simulink model "TosAeb"  (R2021a)
settings: Solver=FixedStepDiscrete  StartTime=0.0  StopTime=inf  FixedStep=0.01  RelTol=1e-3
undefined symbols (not defined in the file or in its folder): ACCModSts, AccSts1, AEB_ObjectMovingOrientation, AEB_ObjectMovingState, CN_BTCActive, CN_CurrentGearR, CN_CurrentGearR_DHT, CN_PTCActive, CN_VDCActive, CN_VSC_EspOff, CN_VSC_FCW_AEB_Error, CN_VSC_FCW_AEB_NoDispay, CN_VSC_FCW_AEB_PerfmDgdtn, CN_VSC_FCW_AEB_TempUnAvlbl, CN_VSC_VehStandstill, CNaccelAvoidMax, CNaccelAvoidMin, CNbrakePedalPress, CNcomfortStrLeftAccelMax, CNcomfortStrLeftAccelMin, CNcomfortStrRightAccelMax, CNcomfortStrRightAccelMin, CNDrvDoorOpen, CNDrvModeEco, CNDrvModeNorm, CNDrvModeOther, CNDrvModeSport, CNDrvSBRreminder, CNNull, CNobjInvalidID, CNPassDoorOpen, CNPassSBRreminder, CNPEBSAccelNegInf, CNPEBSAccelNull, CNPEBSTimeInf, CNPEBSTimeNull, CNSTOPMAX, CNSTOPNULL, CNsubjWidth, CNSwtOFF, CNSwtON, CNTTCMAX, CNTTCNULL, CNVehConfigAEBCycNone, CNVehConfigAEBNone, CNVehConfigAEBPedNone, CNVehConfigFCWNone, CNVehConfigJANone, CollisionSeverity, FALSE, FCWSnvty, JACycObjCalcInfoDefaultParams, JAPedObjCalcInfoDefaultParams, JAVehObjCalcInfoDefaultParams, latCycObjCalcInfoDefaultParams, latPedObjCalcInfoDefaultParams, longObjCalcInfoDefaultParams, ModSts, movingStatus, ObjectType ... (+1112)
libraries: simulink x3031, AEBSFunctionLib x50, Ta_Library x33, AEBSStateMachineLib x23, Mylibrary x12, simulink_extras x7, ObjSelect_lib x3, dspstat3 x2
(part 20 of 102)

== system TosAeb/FcwAebJa_Step_sys/ALG_AEBSystem/ALG_AEBOutput/AEBS_DVRInfoCalc/AEB_LATCYC_DVR_INFO/Subsystem ==
  latCycTgtCalcInfo : Inport
  Action Port : ActionPort  ActionPortLabel=else
  AEBObjStateMap : Subsystem
  Bus Creator : BusCreator  Inputs=12
  Bus Selector2 : BusSelector  OutputSignals=latCycObjCalcInfo.latCycObjRawInfo.ObjectID,latCycObjCalcInfo.latCycObjRawInfo.ObjectTypeCameraConfirmed,latCycObjCalcInfo.latCycObjRawInfo.ObjectMovingState,latCycObjCalcInfo.latCycObjRawInfo.ObjectMovingOrientation,latCycObjCalcInfo.latCycObjRawInfo.dx,latCycObjCalcInfo.latCycObjRawInfo.dy,latCycObjCalcInfo.latCycObjRawInfo.vx,latCycObjCalcInfo.latCycObjRawInfo.vy,latCycObjCalcInfo.latCycObjRawInfo.ax,latCycObjCalcInfo.latCycObjRawInfo.ay,latCycObjCalcInfo.latCycObjRawInfo.ObjectExistProb,latCycObjCalcInfo.latCycObjRawInfo.ObjectIsObstacleProb,latCycObjCalcInfo.latCycObjRawInfo.ObjectLifeCycle
  Signal Conversion{26..37} (12 alike) : SignalConversion  OverrideOpt=off
  AEB_LATCYC_DVR_INFO : Outport
  wires:
  Signal Conversion30/1 -> Bus Creator/5  "CyclistObjDy"
  Signal Conversion28/1 -> Bus Creator/3  "CyclistObjState"
  Signal Conversion26/1 -> Bus Creator/1  "CyclistObjID"
  Signal Conversion36/1 -> Bus Creator/11  "CyclistObjObstcl"
  Signal Conversion35/1 -> Bus Creator/10  "CyclistObjExist"
  Bus Selector2/3 -> AEBObjStateMap/1(ObjectMovingState)  "<ObjectMovingState>"
  Bus Selector2/4 -> AEBObjStateMap/2(ObjectMovingOrientation)  "<ObjectMovingOrientation>"
  Signal Conversion33/1 -> Bus Creator/8  "CyclistObjAx"
  AEBObjStateMap/1(AEBObjState) -> Signal Conversion28/1
  Bus Selector2/5 -> Signal Conversion29/1  "<dx>"
  Bus Selector2/6 -> Signal Conversion30/1  "<dy>"
  latCycTgtCalcInfo/1 -> Bus Selector2/1
  Bus Selector2/7 -> Signal Conversion31/1  "<vx>"
  Bus Selector2/8 -> Signal Conversion32/1  "<vy>"
  Bus Selector2/9 -> Signal Conversion33/1  "<ax>"
  Bus Selector2/12 -> Signal Conversion36/1  "<ObjectIsObstacleProb>"
  Bus Selector2/1 -> Signal Conversion26/1  "<ObjectID>"
  Signal Conversion31/1 -> Bus Creator/6  "CyclistObjVx"
  Bus Creator/1 -> AEB_LATCYC_DVR_INFO/1
  Signal Conversion29/1 -> Bus Creator/4  "CyclistObjDx"
  Bus Selector2/11 -> Signal Conversion35/1  "<ObjectExistProb>"
  Signal Conversion37/1 -> Bus Creator/12  "CyclistObjCntAlive"
  Bus Selector2/2 -> Signal Conversion27/1  "<ObjectTypeCameraConfirmed>"
  Signal Conversion27/1 -> Bus Creator/2  "CyclistObjVideoCfmd"
  Bus Selector2/13 -> Signal Conversion37/1  "<ObjectLifeCycle>"
  Bus Selector2/10 -> Signal Conversion34/1  "<ay>"
  Signal Conversion34/1 -> Bus Creator/9  "CyclistObjAy"
  Signal Conversion32/1 -> Bus Creator/7  "CyclistObjVy"

== system TosAeb/FcwAebJa_Step_sys/ALG_AEBSystem/ALG_AEBOutput/AEBS_DVRInfoCalc/AEB_LATPED_DVR_INFO/If Action Subsystem ==
  Action Port : ActionPort  ActionPortLabel=if(u1)
  Bus Creator : BusCreator  Inputs=12
  Constant{,1..11} (12 alike) : Constant  Value=0
  Data Type Conversion{,1..11} (12 alike) : DataTypeConversion
  AEB_LATPED_DVR_INFO : Outport
  wires:
  Constant8/1 -> Data Type Conversion8/1
  Constant9/1 -> Data Type Conversion9/1
  Constant11/1 -> Data Type Conversion11/1
  Constant10/1 -> Data Type Conversion10/1
  Constant4/1 -> Data Type Conversion4/1
  Constant5/1 -> Data Type Conversion5/1
  Constant7/1 -> Data Type Conversion7/1
  Constant6/1 -> Data Type Conversion6/1
  Constant2/1 -> Data Type Conversion2/1
  Constant3/1 -> Data Type Conversion3/1
  Constant1/1 -> Data Type Conversion1/1
  Constant/1 -> Data Type Conversion/1
  Data Type Conversion6/1 -> Bus Creator/7  "PedObjVy"
  Data Type Conversion1/1 -> Bus Creator/2  "PedObjVideoCfmd"
  Bus Creator/1 -> AEB_LATPED_DVR_INFO/1
  Data Type Conversion2/1 -> Bus Creator/3  "PedObjState"
  Data Type Conversion11/1 -> Bus Creator/12  "PedObjCntAlive"
  Data Type Conversion4/1 -> Bus Creator/5  "PedObjDy"
  Data Type Conversion7/1 -> Bus Creator/8  "PedObjAx"
  Data Type Conversion3/1 -> Bus Creator/4  "PedObjDx"
  Data Type Conversion/1 -> Bus Creator/1  "PedObjID"
  Data Type Conversion10/1 -> Bus Creator/11  "PedObjObstcl"
  Data Type Conversion8/1 -> Bus Creator/9  "PedObjAy"
  Data Type Conversion9/1 -> Bus Creator/10  "PedObjExist"
  Data Type Conversion5/1 -> Bus Creator/6  "PedObjVx"

== system TosAeb/FcwAebJa_Step_sys/ALG_AEBSystem/ALG_AEBOutput/AEBS_DVRInfoCalc/AEB_LATPED_DVR_INFO/Subsystem ==
  latPedTgtCalcInfo : Inport
  Action Port : ActionPort  ActionPortLabel=else
  AEBObjStateMap : Subsystem
  Bus Creator : BusCreator  Inputs=12
  Bus Selector1 : BusSelector  OutputSignals=latPedObjCalcInfo.latPedObjRawInfo.ObjectID,latPedObjCalcInfo.latPedObjRawInfo.ObjectTypeCameraConfirmed,latPedObjCalcInfo.latPedObjRawInfo.ObjectMovingState,latPedObjCalcInfo.latPedObjRawInfo.ObjectMovingOrientation,latPedObjCalcInfo.latPedObjRawInfo.dx,latPedObjCalcInfo.latPedObjRawInfo.dy,latPedObjCalcInfo.latPedObjRawInfo.vx,latPedObjCalcInfo.latPedObjRawInfo.vy,latPedObjCalcInfo.latPedObjRawInfo.ax,latPedObjCalcInfo.latPedObjRawInfo.ay,latPedObjCalcInfo.latPedObjRawInfo.ObjectExistProb,latPedObjCalcInfo.latPedObjRawInfo.ObjectIsObstacleProb,latPedObjCalcInfo.latPedObjRawInfo.ObjectLifeCycle
  Signal Conversion{14..25} (12 alike) : SignalConversion  OverrideOpt=off
  AEB_LATPED_DVR_INFO : Outport
  wires:
  Bus Selector1/6 -> Signal Conversion18/1  "<dy>"
  Bus Selector1/7 -> Signal Conversion19/1  "<vx>"
  Bus Selector1/2 -> Signal Conversion15/1  "<ObjectTypeCameraConfirmed>"
  Bus Selector1/3 -> AEBObjStateMap/1(ObjectMovingState)  "<ObjectMovingState>"
  Bus Selector1/8 -> Signal Conversion20/1  "<vy>"
  Bus Selector1/10 -> Signal Conversion22/1  "<ay>"
  Bus Selector1/9 -> Signal Conversion21/1  "<ax>"
  Bus Selector1/4 -> AEBObjStateMap/2(ObjectMovingOrientation)  "<ObjectMovingOrientation>"
  Bus Creator/1 -> AEB_LATPED_DVR_INFO/1
  Signal Conversion20/1 -> Bus Creator/7  "PedObjVy"
  Signal Conversion19/1 -> Bus Creator/6  "PedObjVx"
  Signal Conversion23/1 -> Bus Creator/10  "PedObjExist"
  Bus Selector1/13 -> Signal Conversion25/1  "<ObjectLifeCycle>"
  Signal Conversion21/1 -> Bus Creator/8  "PedObjAx"
  Bus Selector1/11 -> Signal Conversion23/1  "<ObjectExistProb>"
  Signal Conversion22/1 -> Bus Creator/9  "PedObjAy"
  Signal Conversion24/1 -> Bus Creator/11  "PedObjObstcl"
  Signal Conversion14/1 -> Bus Creator/1  "PedObjID"
  Signal Conversion15/1 -> Bus Creator/2  "PedObjVideoCfmd"
  latPedTgtCalcInfo/1 -> Bus Selector1/1
  Bus Selector1/12 -> Signal Conversion24/1  "<ObjectIsObstacleProb>"
  Signal Conversion25/1 -> Bus Creator/12  "PedObjCntAlive"
  Signal Conversion17/1 -> Bus Creator/4  "PedObjDx"
  AEBObjStateMap/1(AEBObjState) -> Signal Conversion16/1
  Signal Conversion18/1 -> Bus Creator/5  "PedObjDy"
  Bus Selector1/5 -> Signal Conversion17/1  "<dx>"
  Signal Conversion16/1 -> Bus Creator/3  "PedObjState"
  Bus Selector1/1 -> Signal Conversion14/1  "<ObjectID>"

== system TosAeb/FcwAebJa_Step_sys/ALG_AEBSystem/ALG_AEBOutput/AEBS_DVRInfoCalc/AEB_LON_DVR_INFO/If Action Subsystem ==
  longObjCalcInfo : Inport
  Action Port : ActionPort  ActionPortLabel=else
  AEBObjStateMap : Subsystem
  Bus Creator : BusCreator  Inputs=14
  Bus Selector : BusSelector  OutputSignals=LongObjRawInfo.ObjectID,LongObjRawInfo.ObjectTypeCameraConfirmed,LongObjRawInfo.ObjectMovingState,LongObjRawInfo.ObjectMovingOrientation,LongObjRawInfo.dx,LongObjRawInfo.dy,LongObjRawInfo.vx,LongObjRawInfo.vy,LongObjRawInfo.ax,LongObjRawInfo.ay,LongObjRawInfo.ObjectExistProb,LongObjRawInfo.ObjectIsObstacleProb,LongObjRawInfo.ObjectLifeCycle,overlapRatio,probEscape
  Signal Conversion{,1..13} (14 alike) : SignalConversion  OverrideOpt=off
  AEB_LON_DVR_INFO : Outport
  wires:
  Bus Creator/1 -> AEB_LON_DVR_INFO/1
  Bus Selector/6 -> Signal Conversion4/1  "<dy>"
  Signal Conversion3/1 -> Bus Creator/4  "AEBObjDx"
  Signal Conversion/1 -> Bus Creator/1  "AEBObjID"
  longObjCalcInfo/1 -> Bus Selector/1
  Signal Conversion10/1 -> Bus Creator/11  "AEBObjObstcl"
  Signal Conversion7/1 -> Bus Creator/8  "AEBObjAx"
  Bus Selector/13 -> Signal Conversion11/1  "<ObjectLifeCycle>"
  Bus Selector/15 -> Signal Conversion13/1  "<probEscape>"
  AEBObjStateMap/1(AEBObjState) -> Signal Conversion2/1
  Bus Selector/8 -> Signal Conversion6/1  "<vy>"
  Signal Conversion2/1 -> Bus Creator/3  "AEBObjState"
  Signal Conversion8/1 -> Bus Creator/9  "AEBObjAy"
  Bus Selector/14 -> Signal Conversion12/1  "<overlapRatio>"
  Bus Selector/3 -> AEBObjStateMap/1(ObjectMovingState)  "<ObjectMovingState>"
  Bus Selector/2 -> Signal Conversion1/1  "<ObjectTypeCameraConfirmed>"
  Signal Conversion9/1 -> Bus Creator/10  "AEBObjExist"
  Bus Selector/1 -> Signal Conversion/1  "<ObjectID>"
  Signal Conversion13/1 -> Bus Creator/14  "AEBObjDrvby"
  Signal Conversion5/1 -> Bus Creator/6  "AEBObjVx"
  Signal Conversion11/1 -> Bus Creator/12  "AEBObjCntAlive"
  Bus Selector/5 -> Signal Conversion3/1  "<dx>"
  Signal Conversion12/1 -> Bus Creator/13  "AEBObjOverlap"
  Signal Conversion1/1 -> Bus Creator/2  "AEBObjVideoCfmd"
  Signal Conversion6/1 -> Bus Creator/7  "AEBObjVy"
  Signal Conversion4/1 -> Bus Creator/5  "AEBObjDy"
  Bus Selector/10 -> Signal Conversion8/1  "<ay>"
  Bus Selector/4 -> AEBObjStateMap/2(ObjectMovingOrientation)  "<ObjectMovingOrientation>"
  Bus Selector/7 -> Signal Conversion5/1  "<vx>"
  Bus Selector/12 -> Signal Conversion10/1  "<ObjectIsObstacleProb>"
  Bus Selector/9 -> Signal Conversion7/1  "<ax>"
  Bus Selector/11 -> Signal Conversion9/1  "<ObjectExistProb>"

== system TosAeb/FcwAebJa_Step_sys/ALG_AEBSystem/ALG_AEBOutput/AEBS_DVRInfoCalc/AEB_LON_DVR_INFO/If Action Subsystem1 ==
  Action Port : ActionPort  ActionPortLabel=if(u1)
  Bus Creator : BusCreator  Inputs=14
  Constant{,1..13} (14 alike) : Constant  Value=0
  Data Type Conversion{,1..13} (14 alike) : DataTypeConversion
  AEB_LON_DVR_INFO : Outport
  wires:
  Constant12/1 -> Data Type Conversion12/1
  Constant13/1 -> Data Type Conversion13/1
  Constant7/1 -> Data Type Conversion7/1
  Constant8/1 -> Data Type Conversion8/1
  Constant11/1 -> Data Type Conversion11/1
  Constant10/1 -> Data Type Conversion10/1
  Constant9/1 -> Data Type Conversion9/1
  Constant4/1 -> Data Type Conversion4/1
  Constant5/1 -> Data Type Conversion5/1
  Constant6/1 -> Data Type Conversion6/1
  Constant3/1 -> Data Type Conversion3/1
  Constant2/1 -> Data Type Conversion2/1
  Constant1/1 -> Data Type Conversion1/1
  Constant/1 -> Data Type Conversion/1
  Data Type Conversion6/1 -> Bus Creator/7  "AEBObjVy"
  Data Type Conversion4/1 -> Bus Creator/5  "AEBObjDy"
  Data Type Conversion1/1 -> Bus Creator/2  "AEBObjVideoCfmd"
  Data Type Conversion5/1 -> Bus Creator/6  "AEBObjVx"
  Data Type Conversion11/1 -> Bus Creator/12  "AEBObjCntAlive"
  Data Type Conversion12/1 -> Bus Creator/13  "AEBObjOverlap"
  Data Type Conversion13/1 -> Bus Creator/14  "AEBObjDrvby"
  Data Type Conversion2/1 -> Bus Creator/3  "AEBObjState"
  Data Type Conversion9/1 -> Bus Creator/10  "AEBObjExist"
  Data Type Conversion8/1 -> Bus Creator/9  "AEBObjAy"
  Data Type Conversion7/1 -> Bus Creator/8  "AEBObjAx"
  Data Type Conversion10/1 -> Bus Creator/11  "AEBObjObstcl"
  Data Type Conversion3/1 -> Bus Creator/4  "AEBObjDx"
  Data Type Conversion/1 -> Bus Creator/1  "AEBObjID"
  Bus Creator/1 -> AEB_LON_DVR_INFO/1

== system TosAeb/FcwAebJa_Step_sys/ALG_AEBSystem/ALG_AEBOutput/AEBS_IPHUTInfoCalc/AR_HUD_InfoMap/AEB_Cyc_collisionprob ==
  cycPrefillFuncImpArb : Inport
  cycAEBpartFuncImpArb : Inport  Port=2
  cycAEBfullFuncImpArb : Inport  Port=3
  Compare To Constant5 : simulink/Logic and Bit Operations/Compare To Constant  relop===  const=ModSts.ActiveState  OutDataTypeStr=boolean
  Compare To Constant6 : simulink/Logic and Bit Operations/Compare To Constant  relop===  const=ModSts.ActiveState  OutDataTypeStr=boolean
  Compare To Constant7 : simulink/Logic and Bit Operations/Compare To Constant  relop===  const=ModSts.ActiveState  OutDataTypeStr=boolean
  Logical Operator1 : Logic  Operator=OR  Inputs=3  AllPortsSameDT=off  OutDataTypeStr=boolean
  AEB_Cyc_collisionprob : Outport
  wires:
  Compare To Constant5/1 -> Logical Operator1/1
  cycAEBfullFuncImpArb/1 -> Compare To Constant7/1
  cycAEBpartFuncImpArb/1 -> Compare To Constant6/1
  Compare To Constant6/1 -> Logical Operator1/2
  cycPrefillFuncImpArb/1 -> Compare To Constant5/1
  Logical Operator1/1 -> AEB_Cyc_collisionprob/1
  Compare To Constant7/1 -> Logical Operator1/3

== system TosAeb/FcwAebJa_Step_sys/ALG_AEBSystem/ALG_AEBOutput/AEBS_IPHUTInfoCalc/AR_HUD_InfoMap/AEB_Ped_collisionprob ==
  pedPreWarningFuncImpArb : Inport
  pedPrefillFuncImpArb : Inport  Port=2
  pedABAFuncImpArb : Inport  Port=3
  pedAEBfullFuncImpArb : Inport  Port=4
  Compare To Constant{1..4} (4 alike) : simulink/Logic and Bit Operations/Compare To Constant  relop===  const=ModSts.ActiveState  OutDataTypeStr=boolean
  Logical Operator : Logic  Operator=OR  Inputs=4  AllPortsSameDT=off  OutDataTypeStr=boolean
  AEB_Ped_collisionprob : Outport
  wires:
  pedABAFuncImpArb/1 -> Compare To Constant2/1
  pedPrefillFuncImpArb/1 -> Compare To Constant1/1
  Compare To Constant3/1 -> Logical Operator/4
  Compare To Constant1/1 -> Logical Operator/2
  pedAEBfullFuncImpArb/1 -> Compare To Constant3/1
  pedPreWarningFuncImpArb/1 -> Compare To Constant4/1
  Logical Operator/1 -> AEB_Ped_collisionprob/1
  Compare To Constant4/1 -> Logical Operator/1
  Compare To Constant2/1 -> Logical Operator/3

== system TosAeb/AEB_Objsel/TA_InfoRebuild/TAObjInfoCalc/For Each Subsystem/Info_rebuild/Road_relative/LineInfo_Process ==
  Road_Info : Inport
  Bus Selector : BusSelector  OutputSignals=lineData
  LineInfo_Process_Left : Subsystem
  LineInfo_Process_Right : Subsystem
  Selector : Selector  IndexMode=Zero-based  InputPortWidth=4  IndexOptions=Index vector (dialog)  Indices=[0]  OutputSizes=1
  Selector1 : Selector  IndexMode=Zero-based  InputPortWidth=4  IndexOptions=Index vector (dialog)  Indices=[1]  OutputSizes=1
  LeftLine_Info : Outport
  RightLine_Info : Outport  Port=2
  wires:
  Bus Selector/1 -> Selector1/1, Selector/1  "<lineData>"
  LineInfo_Process_Left/1(LeftLine_Info) -> LeftLine_Info/1
  Selector/1 -> LineInfo_Process_Left/1(Leftline_Data)
  Road_Info/1 -> Bus Selector/1
  LineInfo_Process_Right/1(RightLine_Info) -> RightLine_Info/1
  Selector1/1 -> LineInfo_Process_Right/1(Rightline_Data)

== system TosAeb/AEB_Objsel/TA_InfoRebuild/TAObjInfoCalc/For Each Subsystem/Info_rebuild/Road_relative/RebuildInfo_Process ==
  Obj_Infomation : Inport
  LeftLine_Info : Inport  Port=2
  RightLine_Info : Inport  Port=3
  If : If
  If Action Subsystem : Subsystem  TreatAsAtomicUnit=on
  If Action Subsystem1 : Subsystem  TreatAsAtomicUnit=on
  Merge : Merge
  Merge1 : Merge
  Merge2 : Merge
  Subsystem : Subsystem
  objLatDist2LaneCenter : Outport
  objLatDist2EgoCenter : Outport  Port=2
  objPosition : Outport  Port=3
  wires:
  Subsystem/1(if_judge) -> If/1
  If/1 -> If Action Subsystem/Action
  If/2 -> If Action Subsystem1/Action
  Obj_Infomation/1 -> If Action Subsystem1/1(Obj_Infomation), Subsystem/1(Obj_Infomation)
  LeftLine_Info/1 -> If Action Subsystem1/2(LeftLine_Info)
  RightLine_Info/1 -> If Action Subsystem1/3(RightLine_Info)
  If Action Subsystem/1(objLatDist2LaneCenter) -> Merge/1
  Merge/1 -> objLatDist2LaneCenter/1
  If Action Subsystem1/1(objLatDist2LaneCenter) -> Merge/2
  If Action Subsystem/2(objLatDist2EgoCenter) -> Merge1/1
  If Action Subsystem1/2(objLatDist2EgoCenter) -> Merge1/2
  If Action Subsystem/3(objPosition) -> Merge2/1
  If Action Subsystem1/3(objPosition) -> Merge2/2
  Merge1/1 -> objLatDist2EgoCenter/1
  Merge2/1 -> objPosition/1

== system TosAeb/AEB_Objsel/TA_Processor/AEB_ObjSelect/TargetInfoCheck/Data_precess/TA_EffectCalculate/Obj_FilterFlgCalculate ==
  Utility : Inport
  Overlap_Current : Inport  Port=2
  Overlap_Prediction : Inport  Port=3
  PahEstimn : Inport  Port=4
  TA_ObjInfo : Inport  Port=5
  VehSelfData : Inport  Port=6
  JA_ObjFilter : Subsystem
  Lat_ObjFilter : Subsystem
  Long_ObjFilter : Subsystem
  WeightCoef_Cal : Subsystem
  Overlap_Flg : Outport
  Boudingbox_Calculate : Outport  Port=2
  Obj_Coef : Outport  Port=3
  TTI : Outport  Port=4
  LatObj_Valid : Outport  Port=5
  Lat_ObjIntersect : Outport  Port=6
  Lat_TTR : Outport  Port=7
  JA_TTR : Outport  Port=8
  Meas_WeightCoef_1 : Outport  Port=9
  Meas_WeightCoef_2 : Outport  Port=10
  wires:
  TA_ObjInfo/1 -> [TA_ObjInfo], Long_ObjFilter/2(TA_ObjInfo)
  VehSelfData/1 -> [VehSelfData], Long_ObjFilter/3(VehSelfData)
  Utility/1 -> [Utility], WeightCoef_Cal/2(Utility)
  Overlap_Current/1 -> Overlap_Flg/1, WeightCoef_Cal/1(Overlap_Current)  "Overlap_Current"
  Overlap_Prediction/1 -> WeightCoef_Cal/3(Overlap_Prediction)  "Overlap_Prediction"
  PahEstimn/1 -> [PahEstimn], Long_ObjFilter/4(" PahEstimn")
  Long_ObjFilter/1(Boudingbox_Calculate) -> Boudingbox_Calculate/1
  Long_ObjFilter/2(Obj_Coef) -> Obj_Coef/1
  Long_ObjFilter/3(TTI) -> TTI/1
  [VehSelfData] -> WeightCoef_Cal/4(VehSelfData)
  [TA_ObjInfo] -> Lat_ObjFilter/2(TA_ObjInfo)
  Lat_ObjFilter/3(Lat_TTR) -> Lat_TTR/1
  Lat_ObjFilter/1(LatObj_Valid) -> LatObj_Valid/1
  Lat_ObjFilter/2(Lat_ObjIntersect) -> Lat_ObjIntersect/1
  [Utility] -> Lat_ObjFilter/1(Utility)
  [VehSelfData] -> Lat_ObjFilter/3(VehSelfData)
  [TA_ObjInfo] -> JA_ObjFilter/1(TA_ObjInfo)
  [PahEstimn] -> JA_ObjFilter/2(PahEstimn)
  [VehSelfData] -> JA_ObjFilter/3(VehSelfData)
  JA_ObjFilter/1(ObjColliInfo) -> JA_TTR/1
  WeightCoef_Cal/1(Obj_WeightCoef) -> Meas_WeightCoef_1/1, Long_ObjFilter/1(Obj_WeightCoef)
  Long_ObjFilter/4(Long_ObjWeightCoef) -> Meas_WeightCoef_2/1

== system TosAeb/AEB_Objsel/TA_Processor/AEB_ObjSelect/TargetInfoCheck/Data_precess/TA_EffectCalculate/ValideateObj ==
  TA_ObsInfo : Inport
  VehSelfData : Inport  Port=2
  PathEstimn : Inport  Port=3
  ObjPos_Calculate : Subsystem
  ValidObj_PreProcess : Subsystem
  Utility : Outport
  Overlap_Current : Outport  Port=2
  Overlap_Prediction : Outport  Port=3
  wires:
  VehSelfData/1 -> ValidObj_PreProcess/2(VehSelfData)
  ObjPos_Calculate/2(Overlap_Current) -> Overlap_Current/1
  ObjPos_Calculate/3(Overlap_Prediction) -> Overlap_Prediction/1
  TA_ObsInfo/1 -> ValidObj_PreProcess/1(TA_ObjInfo), ObjPos_Calculate/1(TA_ObsInfo)
  PathEstimn/1 -> ObjPos_Calculate/3(PathEstimn)
  ValidObj_PreProcess/1(Obj_Enable) -> ObjPos_Calculate/2(Obj_Enable)
  ObjPos_Calculate/1(Utility) -> Utility/1

== system TosAeb/AEB_Objsel/TA_Processor/AEB_ObjSelect/TargetSelect/Lat_ObjSelect/AEB_LatObj/AEB_LatTAelse ==
  LatObj_Valid : Inport
  TA_ObjInfo : Inport  Port=2
  Lat_ObjIntersect : Inport  Port=3
  Lat_TTR : Inport  Port=4
  Action Port : ActionPort  ActionPortLabel=else
  Bus Selector : BusSelector  OutputSignals=TA_ObjID
  Constant : Constant  Value=zeros(10,1)  OutDataTypeStr=uint8
  Constant1 : Constant  Value=zeros(10,1)  OutDataTypeStr=uint8
  Lat_TASelect : Stateflow chart  TreatAsAtomicUnit=on
  Saturation : Saturate  UpperLimit=32  LowerLimit=0
  Sort : dspstat3/Sort  otype=Index  dir=Ascending  sortAlg=Quick sort  roundingMode=Floor  overflowMode=off  accumDataTypeStr=Inherit: Same as product output  accumLastDataTypeStr=Inherit: Same as product output
      settings: SourceProductBaseCode=DS
  Sum of Elements : Sum  Inputs=+
  num : Outport
  ID : Outport  Port=2
  wires:
  LatObj_Valid/1 -> Lat_TASelect/1(Obj_Valid)
  TA_ObjInfo/1 -> Bus Selector/1
  Bus Selector/1 -> Lat_TASelect/2(Obj_ID)  "<TA_ObjID>"
  Lat_TTR/1 -> Sort/1
  Lat_ObjIntersect/1 -> Lat_TASelect/4(Lat_ObjIntersect), Sum of Elements/1
  Sum of Elements/1 -> Lat_TASelect/3(sumLat_ObjFlg)
  Lat_TASelect/1(num) -> Saturation/1
  Saturation/1 -> num/1
  Lat_TASelect/2(ID) -> ID/1
  Constant/1 -> Lat_TASelect/6(Init_ID)
  Constant1/1 -> Lat_TASelect/7(Init_Num)
  Sort/1 -> Lat_TASelect/5(Sort)

== system TosAeb/AEB_Objsel/TA_Processor/AEB_ObjSelect/TargetSelect/Lat_ObjSelect/AEB_LatObj/AEB_LatTAif ==
  LatObj_Valid : Inport
  TA_ObjInfo : Inport  Port=2
  Action Port : ActionPort  ActionPortLabel=if(u1 > 0)
  Bus Selector : BusSelector  OutputSignals=TA_ObjID
  Constant : Constant  Value=zeros(10,1)  OutDataTypeStr=uint8
  Constant1 : Constant  Value=zeros(10,1)  OutDataTypeStr=uint8
  Lat_TASelect : Stateflow chart  TreatAsAtomicUnit=on
  Saturation : Saturate  UpperLimit=32  LowerLimit=0
  num : Outport
  ID : Outport  Port=2
  wires:
  LatObj_Valid/1 -> Lat_TASelect/1(Obj_Valid)
  TA_ObjInfo/1 -> Bus Selector/1
  Bus Selector/1 -> Lat_TASelect/2(Obj_ID)  "<TA_ObjID>"
  Constant/1 -> Lat_TASelect/3(Init_Num)
  Constant1/1 -> Lat_TASelect/4(Init_ID)
  Lat_TASelect/1(num) -> Saturation/1
  Saturation/1 -> num/1
  Lat_TASelect/2(ID) -> ID/1

== system TosAeb/AEB_Objsel/TA_Processor/AEB_ObjSelect/TargetSelect/Lat_ObjSelect/AEB_LatObj/ObjNumValid ==
  Lat_ObjNum : Inport
  Compare To Constant : simulink/Logic and Bit Operations/Compare To Constant  relop=<=  const=10  OutDataTypeStr=boolean
  Data Type Conversion : DataTypeConversion
  Obj_NumBelow : Outport
  wires:
  Lat_ObjNum/1 -> Compare To Constant/1
  Compare To Constant/1 -> Data Type Conversion/1
  Data Type Conversion/1 -> Obj_NumBelow/1

== system TosAeb/AEB_Objsel/TA_Processor/AEB_ObjSelect/TargetSelect/Long_ObjSelect/Obj_IDConfirm/Robust ==
  ValidTarget_ID : Inport
  Num_IN : Inport  Port=2
  Add : Sum
  Add1 : Sum
  Compare To Constant : simulink/Logic and Bit Operations/Compare To Constant  relop=>=  const=0.5  OutDataTypeStr=boolean
  Compare To Constant1 : simulink/Logic and Bit Operations/Compare To Constant  relop=>=  const=5  OutDataTypeStr=boolean
  Compare To Constant2 : simulink/Logic and Bit Operations/Compare To Constant  relop===  const=0  OutDataTypeStr=boolean
  Compare To Constant3 : simulink/Logic and Bit Operations/Compare To Constant  relop===  const=32  OutDataTypeStr=boolean
  Compare To Constant4 : simulink/Logic and Bit Operations/Compare To Constant  relop===  const=32  OutDataTypeStr=boolean
  Compare To Constant5 : simulink/Logic and Bit Operations/Compare To Constant  relop===  const=255  OutDataTypeStr=boolean
  Constant : Constant  Value=0  OutDataTypeStr=uint8
  Constant1 : Constant  OutDataTypeStr=uint8
  Constant2 : Constant  Value=0  OutDataTypeStr=uint8
  Constant3 : Constant  OutDataTypeStr=uint8
  Data Type Conversion : DataTypeConversion  OutDataTypeStr=uint8
  Logical Operator : Logic  AllPortsSameDT=off  OutDataTypeStr=boolean
  Logical Operator1 : Logic  Inputs=3  AllPortsSameDT=off  OutDataTypeStr=boolean
  Logical Operator2 : Logic  AllPortsSameDT=off  OutDataTypeStr=boolean
  Logical Operator3 : Logic  Operator=NOT  AllPortsSameDT=off  OutDataTypeStr=boolean
  Relational Operator : RelationalOperator  Operator=~=  OutDataTypeStr=boolean  RndMeth=Simplest
  Relational Operator1 : RelationalOperator  Operator=~=  OutDataTypeStr=boolean  RndMeth=Simplest
  Saturation : Saturate  UpperLimit=254  LowerLimit=0
  Saturation1 : Saturate  UpperLimit=254  LowerLimit=0
  Switch{,2,4..5} (4 alike) : Switch  Criteria=u2 ~= 0
  Switch1 : Switch  Criteria=u2 > Threshold
  Switch3 : Switch  Criteria=u2 > Threshold
  Unit Delay{,1..3} (4 alike) : UnitDelay  SampleTime=-1  HasFrameUpgradeWarning=on
  ID : Outport
  Num : Outport  Port=2
  wires:
  Switch/1 -> ID/1, Unit Delay/1
  ValidTarget_ID/1 -> [A], Switch/1, Relational Operator/1
  Relational Operator/1 -> Switch1/2
  Unit Delay/1 -> Relational Operator/2, Switch/3
  Constant/1 -> Switch1/3
  Unit Delay1/1 -> Add/1
  Constant1/1 -> Add/2
  Switch1/1 -> Saturation/1
  Compare To Constant/1 -> Switch/2
  Saturation/1 -> Compare To Constant/1, Unit Delay1/1
  Add/1 -> Switch1/1
  Compare To Constant3/1 -> Logical Operator/2
  Compare To Constant2/1 -> Logical Operator/1
  Num_IN/1 -> Compare To Constant3/1, Compare To Constant4/1, Switch2/1, Relational Operator1/1, Switch5/1, Switch4/1
  Switch5/1 -> Switch4/3
  Saturation1/1 -> Unit Delay3/1, Compare To Constant1/1
  Switch4/1 -> Data Type Conversion/1
  Constant3/1 -> Add1/2
  Add1/1 -> Switch3/1
  Unit Delay3/1 -> Add1/1
  Constant2/1 -> Switch3/3
  Compare To Constant1/1 -> Switch2/2
  Logical Operator/1 -> Switch4/2
  Relational Operator1/1 -> [b], Logical Operator1/1
  Logical Operator1/1 -> Switch3/2
  Switch3/1 -> Saturation1/1
  Compare To Constant4/1 -> [c], Logical Operator1/2
  [A] -> Compare To Constant5/1
  Compare To Constant5/1 -> Logical Operator1/3
  [c] -> Logical Operator3/1
  Logical Operator3/1 -> Logical Operator2/1
  [b] -> Logical Operator2/2
  Logical Operator2/1 -> Switch5/2
  Switch2/1 -> Switch5/3
  Unit Delay2/1 -> Compare To Constant2/1, Relational Operator1/2, Switch2/3
  Data Type Conversion/1 -> Unit Delay2/1, Num/1

== system TosAeb/AEB_Objsel/TA_Processor/AEB_ObjSelect/TargetSelect/Long_ObjSelect/Obj_IDConfirm/Target_Select_decle ==
  TA_ObjInfo : Inport
  VehSelfData : Inport  Port=2
  Boudingbox_Calculate : Inport  Port=3
  Obj_Coef : Inport  Port=4
  TTI : Inport  Port=5
  AccTgtIdn : Inport  Port=6
  Overlap_Rate : Inport  Port=7
  Bus Selector1 : BusSelector  OutputSignals=VehSelf.ABS_VehicleSpeed,VehSelf.AEB_Intervention_ESP
  Bus Selector2 : BusSelector  OutputSignals=TA_ObjID,TA_ObjVx,TA_ObjState,TA_ObjOrientation,TA_ObjDy,TA_ObjDx,TA_ObjVxAbs
  Constant : Constant  Value=P_TA_PrmForVehSpdStationary
  Constant1 : Constant  Value=P_TA_PrmForObjSpdStationary
  Constant2 : Constant  Value=P_TA_PrmForObjSpdStationary
  Obj_ConfirmFunc : MATLAB Function  TreatAsAtomicUnit=on
  Obj_select : MATLAB Function  TreatAsAtomicUnit=on
  Saturation : Saturate  UpperLimit=32  LowerLimit=1
  Sort : dspstat3/Sort  otype=Index  dir=Ascending  sortAlg=Quick sort  roundingMode=Floor  overflowMode=off  accumDataTypeStr=Inherit: Same as product output  accumLastDataTypeStr=Inherit: Same as product output
      settings: SourceProductBaseCode=DS
  ValidTarget_ID : Outport
  Num : Outport  Port=2
  long_TTC : Outport  Port=3
  wires:
  AccTgtIdn/1 -> Obj_select/13(AccTgtIdn)
  TTI/1 -> Obj_ConfirmFunc/10(TTI)
  Obj_select/2(ID_num) -> Saturation/1
  Bus Selector1/2 -> [AEB_State]  "<AEB_Intervention_ESP>"
  Constant1/1 -> Obj_ConfirmFunc/13(P_TA_PrmForObjSpdStationary)
  Boudingbox_Calculate/1 -> Obj_select/10(Boudingbox_Calculate)
  Obj_Coef/1 -> [Obj_coef], Obj_select/11(Obj_Coef)
  TA_ObjInfo/1 -> Bus Selector2/1
  VehSelfData/1 -> Bus Selector1/1
  Overlap_Rate/1 -> [Overlap_rate], Obj_ConfirmFunc/11(Overlap_Rate)
  Bus Selector1/1 -> [Subj_Vx], Obj_ConfirmFunc/8(Subj_vx)  "<ABS_VehicleSpeed>"
  Bus Selector2/4 -> [Obj_Oreintation], Obj_ConfirmFunc/4(Obj_Orentation)  "<TA_ObjOrientation>"
  Bus Selector2/1 -> Obj_ConfirmFunc/1(Obj_ID)  "<TA_ObjID>"
  Bus Selector2/3 -> [Obj_State], Obj_ConfirmFunc/3(Obj_state)  "<TA_ObjState>"
  Bus Selector2/2 -> [Obj_vx], Obj_ConfirmFunc/2(Obj_vx)  "<TA_ObjVx>"
  Constant/1 -> Obj_ConfirmFunc/12(P_TA_PrmForVehSpdStationary)
  Saturation/1 -> Num/1
  Obj_select/3(long_TTC) -> long_TTC/1
  Obj_select/1(ValidTarget_ID) -> ValidTarget_ID/1
  Bus Selector2/5 -> Obj_ConfirmFunc/5(Obj_dy)  "<TA_ObjDy>"
  Bus Selector2/6 -> Obj_ConfirmFunc/6(Obj_dx)  "<TA_ObjDx>"
  Bus Selector2/7 -> Obj_ConfirmFunc/7(obj_vxabs)  "<TA_ObjVxAbs>"
  Constant2/1 -> Obj_select/15(P_TA_PrmForObjSpdStationary)
  Obj_ConfirmFunc/5(re_TTC) -> [re_TTC]
  [re_TTC] -> Obj_select/12(TTI)
  Obj_ConfirmFunc/4(re_dy) -> [re_dy]
  [re_dy] -> Obj_select/4(dy)
  Obj_ConfirmFunc/3(re_dx) -> Sort/1
  Sort/1 -> [re_dx]
  [re_dx] -> Obj_select/3(sort_dx)
  Obj_ConfirmFunc/1(re_Valid) -> [re_Valid]
  [re_Valid] -> Obj_select/1(re_Valid)
  Obj_ConfirmFunc/2(re_ID) -> [re_ID]
  [re_ID] -> Obj_select/2(Obj_ID)
  [Overlap_rate] -> Obj_select/14(Overlap_Rate)
  [AEB_State] -> Obj_select/9(Veh_AEBFunState)
  [Obj_State] -> Obj_select/6(Obj_state)
  [Obj_Oreintation] -> Obj_select/7(Obj_Orentation)
  [Obj_vx] -> Obj_select/5(Obj_vx)
  [Subj_Vx] -> Obj_select/8(Subj_vx)
  [Obj_coef] -> Obj_ConfirmFunc/9(Obj_Coef)

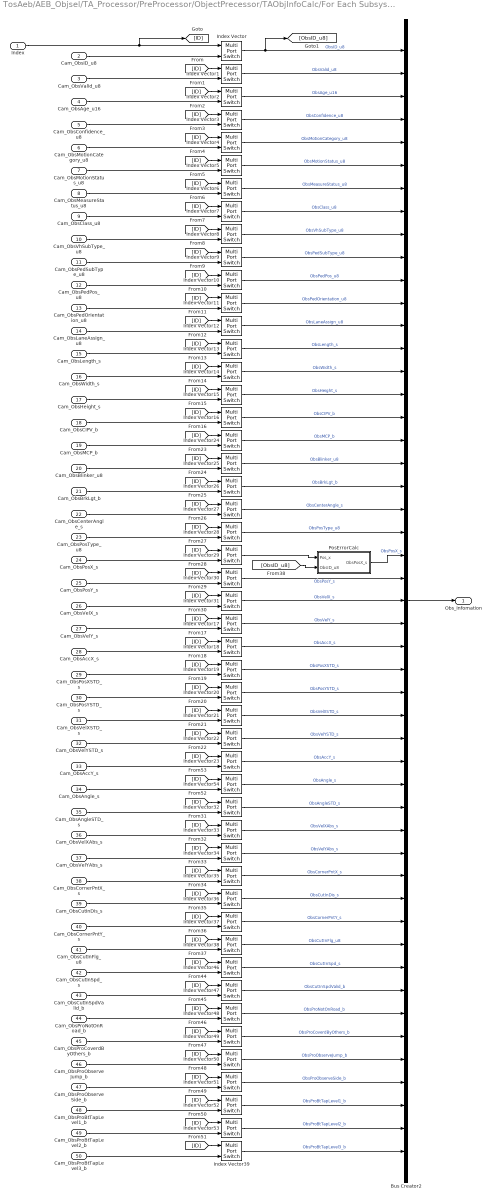
== system TosAeb/AEB_Objsel/TA_Processor/PreProcessor/ObjectPrecessor/TAObjInfoCalc/For Each Subsystem/ForEachInput ==
  Index : Inport
  Cam_ObsID_u8 : Inport  Port=2
  Cam_ObsValid_u8 : Inport  Port=3
  Cam_ObsAge_u16 : Inport  Port=4
  Cam_ObsConfidence_u8 : Inport  Port=5
  Cam_ObsMotionCategory_u8 : Inport  Port=6
  Cam_ObsMotionStatus_u8 : Inport  Port=7
  Cam_ObsMeasureStatus_u8 : Inport  Port=8
  Cam_ObsClass_u8 : Inport  Port=9
  Cam_ObsVhSubType_u8 : Inport  Port=10
  Cam_ObsPedSubType_u8 : Inport  Port=11
  Cam_ObsPedPos_u8 : Inport  Port=12
  Cam_ObsPedOrientation_u8 : Inport  Port=13
  Cam_ObsLaneAssign_u8 : Inport  Port=14
  Cam_ObsLength_s : Inport  Port=15
  Cam_ObsWidth_s : Inport  Port=16
  Cam_ObsHeight_s : Inport  Port=17
  Cam_ObsCIPV_b : Inport  Port=18
  Cam_ObsMCP_b : Inport  Port=19
  Cam_ObsBlinker_u8 : Inport  Port=20
  Cam_ObsBrkLgt_b : Inport  Port=21
  Cam_ObsCenterAngle_s : Inport  Port=22
  Cam_ObsPosType_u8 : Inport  Port=23
  Cam_ObsPosX_s : Inport  Port=24
  Cam_ObsPosY_s : Inport  Port=25
  Cam_ObsVelX_s : Inport  Port=26
  Cam_ObsVelY_s : Inport  Port=27
  Cam_ObsAccX_s : Inport  Port=28
  Cam_ObsPosXSTD_s : Inport  Port=29
  Cam_ObsPosYSTD_s : Inport  Port=30
  Cam_ObsVelXSTD_s : Inport  Port=31
  Cam_ObsVelYSTD_s : Inport  Port=32
  Cam_ObsAccY_s : Inport  Port=33
  Cam_ObsAngle_s : Inport  Port=34
  Cam_ObsAngleSTD_s : Inport  Port=35
  Cam_ObsVelXAbs_s : Inport  Port=36
  Cam_ObsVelYAbs_s : Inport  Port=37
  Cam_ObsCornerPntX_s : Inport  Port=38
  Cam_ObsCutInDis_s : Inport  Port=39
  Cam_ObsCornerPntY_s : Inport  Port=40
  Cam_ObsCutInFlg_u8 : Inport  Port=41
  Cam_ObsCutInSpd_s : Inport  Port=42
  Cam_ObsCutInSpdValid_b : Inport  Port=43
  Cam_ObsProNotOnRoad_b : Inport  Port=44
  Cam_ObsProCoverdByOthers_b : Inport  Port=45
  Cam_ObsProObserveJump_b : Inport  Port=46
  Cam_ObsProObserveSide_b : Inport  Port=47
  Cam_ObsProBtTapLevel1_b : Inport  Port=48
  Cam_ObsProBtTapLevel2_b : Inport  Port=49
  Cam_ObsProBtTapLevel3_b : Inport  Port=50
  Bus Creator2 : BusCreator  Inputs=49
  Index Vector{,1..14,16..32,34..39,46..54} (47 alike) : MultiPortSwitch  DataPortOrder=Zero-based contiguous  Inputs=1  zeroidx=on
  Index Vector15 : MultiPortSwitch  DataPortOrder=Zero-based contiguous  Inputs=1  zeroidx=on  OutDataTypeStr=single
  Index Vector33 : MultiPortSwitch  DataPortOrder=Zero-based contiguous  Inputs=1  zeroidx=on  OutDataTypeStr=single
  PosErrorCalc : Subsystem
  Obs_Infomation : Outport
  wires:
  [ID] -> Index Vector30/1
  [ID] -> Index Vector53/1
  Cam_ObsBlinker_u8/1 -> Index Vector25/2
  Cam_ObsLaneAssign_u8/1 -> Index Vector12/2
  [ID] -> Index Vector12/1
  Cam_ObsPosXSTD_s/1 -> Index Vector19/2
  [ID] -> Index Vector32/1
  Cam_ObsBrkLgt_b/1 -> Index Vector26/2
  Cam_ObsPosYSTD_s/1 -> Index Vector20/2
  Cam_ObsVelYSTD_s/1 -> Index Vector22/2
  Index Vector36/1 -> Bus Creator2/38  "ObsCutInDis_s"
  Cam_ObsVelXAbs_s/1 -> Index Vector33/2
  [ID] -> Index Vector1/1
  Cam_ObsCutInFlg_u8/1 -> Index Vector38/2
  Index Vector18/1 -> Bus Creator2/27  "ObsAccX_s"
  Cam_ObsID_u8/1 -> Index Vector/2
  Cam_ObsProBtTapLevel3_b/1 -> Index Vector39/2
  Cam_ObsProCoverdByOthers_b/1 -> Index Vector49/2
  Cam_ObsAngleSTD_s/1 -> Index Vector32/2
  Index Vector24/1 -> Bus Creator2/18  "ObsMCP_b"
  [ID] -> Index Vector34/1
  Index Vector34/1 -> Bus Creator2/36  "ObsVelYAbs_s"
  Index Vector3/1 -> Bus Creator2/4  "ObsConfidence_u8"
  [ID] -> Index Vector17/1
  Index Vector/1 -> [ObsID_u8], Bus Creator2/1  "ObsID_u8"
  [ID] -> Index Vector26/1
  [ID] -> Index Vector21/1
  Cam_ObsPedPos_u8/1 -> Index Vector10/2
  Cam_ObsCornerPntY_s/1 -> Index Vector37/2
  Bus Creator2/1 -> Obs_Infomation/1
  Index Vector51/1 -> Bus Creator2/46  "ObsProObserveSide_b"
  Index Vector31/1 -> Bus Creator2/25  "ObsVelX_s"
  Cam_ObsMCP_b/1 -> Index Vector24/2
  [ID] -> Index Vector51/1
  Index Vector33/1 -> Bus Creator2/35  "ObsVelXAbs_s"
  Index Vector16/1 -> Bus Creator2/17  "ObsCIPV_b"
  [ID] -> Index Vector8/1
  Index Vector48/1 -> Bus Creator2/43  "ObsProNotOnRoad_b"
  [ID] -> Index Vector50/1
  Cam_ObsAngle_s/1 -> Index Vector54/2
  [ID] -> Index Vector37/1
  [ID] -> Index Vector9/1
  Index Vector53/1 -> Bus Creator2/48  "ObsProBtTapLevel2_b"
  Cam_ObsPosType_u8/1 -> Index Vector28/2
  Cam_ObsAge_u16/1 -> Index Vector2/2
  [ID] -> Index Vector6/1
  Cam_ObsClass_u8/1 -> Index Vector7/2
  Index Vector21/1 -> Bus Creator2/30  "ObsVelXSTD_s"
  Index Vector6/1 -> Bus Creator2/7  "ObsMeasureStatus_u8"
  Index Vector39/1 -> Bus Creator2/49  "ObsProBtTapLevel3_b"
  Cam_ObsPosX_s/1 -> Index Vector29/2
  Index Vector50/1 -> Bus Creator2/45  "ObsProObserveJump_b"
  Cam_ObsHeight_s/1 -> Index Vector15/2
  [ID] -> Index Vector49/1
  Cam_ObsLength_s/1 -> Index Vector13/2
  Index Vector28/1 -> Bus Creator2/22  "ObsPosType_u8"
  [ID] -> Index Vector3/1
  [ID] -> Index Vector38/1
  Cam_ObsMotionCategory_u8/1 -> Index Vector4/2
  Cam_ObsAccX_s/1 -> Index Vector18/2
  [ID] -> Index Vector22/1
  [ID] -> Index Vector2/1
  [ID] -> Index Vector13/1
  [ID] -> Index Vector10/1
  Index Vector47/1 -> Bus Creator2/42  "ObsCutInSpdValid_b"
  Cam_ObsProBtTapLevel2_b/1 -> Index Vector53/2
  Cam_ObsMeasureStatus_u8/1 -> Index Vector6/2
  [ID] -> Index Vector14/1
  Cam_ObsCIPV_b/1 -> Index Vector16/2
  [ID] -> Index Vector16/1
  Cam_ObsPedOrientation_u8/1 -> Index Vector11/2
  Index Vector29/1 -> PosErrorCalc/1(Pos_x)
  Cam_ObsProObserveSide_b/1 -> Index Vector51/2
  Cam_ObsWidth_s/1 -> Index Vector14/2
  Index Vector38/1 -> Bus Creator2/40  "ObsCutInFlg_u8"
  Cam_ObsCenterAngle_s/1 -> Index Vector27/2
  Cam_ObsValid_u8/1 -> Index Vector1/2
  Cam_ObsVelY_s/1 -> Index Vector17/2
  Cam_ObsCutInDis_s/1 -> Index Vector36/2
  Cam_ObsCutInSpd_s/1 -> Index Vector46/2
  Index Vector13/1 -> Bus Creator2/14  "ObsLength_s"
  Cam_ObsProBtTapLevel1_b/1 -> Index Vector52/2
  Cam_ObsVelX_s/1 -> Index Vector31/2
  [ID] -> Index Vector48/1
  [ID] -> Index Vector23/1
  Index Vector8/1 -> Bus Creator2/9  "ObsVhSubType_u8"
  Index Vector23/1 -> Bus Creator2/32  "ObsAccY_s"
  Index Vector49/1 -> Bus Creator2/44  "ObsProCoverdByOthers_b"
  Index Vector5/1 -> Bus Creator2/6  "ObsMotionStatus_u8"
  Cam_ObsProObserveJump_b/1 -> Index Vector50/2
  [ID] -> Index Vector31/1
  Index Vector17/1 -> Bus Creator2/26  "ObsVelY_s"
  [ID] -> Index Vector11/1
  Cam_ObsVhSubType_u8/1 -> Index Vector8/2
  Index Vector10/1 -> Bus Creator2/11  "ObsPedPos_u8"
  Cam_ObsPedSubType_u8/1 -> Index Vector9/2
  Cam_ObsVelXSTD_s/1 -> Index Vector21/2
  Index Vector30/1 -> Bus Creator2/24  "ObsPosY_s"
  [ID] -> Index Vector46/1
  Index Vector20/1 -> Bus Creator2/29  "ObsPosYSTD_s"
  Index Vector1/1 -> Bus Creator2/2  "ObsValid_u8"
  Index Vector14/1 -> Bus Creator2/15  "ObsWidth_s"
  [ID] -> Index Vector19/1
  Cam_ObsPosY_s/1 -> Index Vector30/2
  Index Vector9/1 -> Bus Creator2/10  "ObsPedSubType_u8"
  [ID] -> Index Vector4/1
  [ID] -> Index Vector39/1
  Index Vector46/1 -> Bus Creator2/41  "ObsCutInSpd_s"
  [ID] -> Index Vector20/1
  Index Vector54/1 -> Bus Creator2/33  "ObsAngle_s"
  [ID] -> Index Vector29/1
  Index Vector37/1 -> Bus Creator2/39  "ObsCornerPntY_s"
  Index Vector27/1 -> Bus Creator2/21  "ObsCenterAngle_s"
  [ID] -> Index Vector24/1
  Index Vector22/1 -> Bus Creator2/31  "ObsVelYSTD_s"
  Cam_ObsProNotOnRoad_b/1 -> Index Vector48/2
  [ID] -> Index Vector18/1
  Cam_ObsVelYAbs_s/1 -> Index Vector34/2
  [ID] -> Index Vector15/1
  Index Vector12/1 -> Bus Creator2/13  "ObsLaneAssign_u8"
  Index Vector4/1 -> Bus Creator2/5  "ObsMotionCategory_u8"
  Index Vector7/1 -> Bus Creator2/8  "ObsClass_u8"
  [ID] -> Index Vector54/1
  Index Vector19/1 -> Bus Creator2/28  "ObsPosXSTD_s"
  [ID] -> Index Vector7/1
  [ID] -> Index Vector25/1
  [ID] -> Index Vector28/1
  Index Vector15/1 -> Bus Creator2/16  "ObsHeight_s"
  Index Vector26/1 -> Bus Creator2/20  "ObsBrkLgt_b"
  Cam_ObsCornerPntX_s/1 -> Index Vector35/2
  Index Vector2/1 -> Bus Creator2/3  "ObsAge_u16"
  [ID] -> Index Vector27/1
  [ID] -> Index Vector5/1
  Index Vector25/1 -> Bus Creator2/19  "ObsBlinker_u8"
  [ID] -> Index Vector52/1
  Cam_ObsCutInSpdValid_b/1 -> Index Vector47/2
  Cam_ObsConfidence_u8/1 -> Index Vector3/2
  Index Vector11/1 -> Bus Creator2/12  "ObsPedOrientation_u8"
  Index Vector35/1 -> Bus Creator2/37  "ObsCornerPntX_s"
  [ID] -> Index Vector36/1
  Index Vector52/1 -> Bus Creator2/47  "ObsProBtTapLevel1_b"
  Cam_ObsAccY_s/1 -> Index Vector23/2
  Cam_ObsMotionStatus_u8/1 -> Index Vector5/2
  [ID] -> Index Vector33/1
  [ID] -> Index Vector47/1
  Index Vector32/1 -> Bus Creator2/34  "ObsAngleSTD_s"
  [ID] -> Index Vector35/1
  PosErrorCalc/1(ObsPosX_s) -> Bus Creator2/23  "ObsPosX_s"
  [ObsID_u8] -> PosErrorCalc/2(ObsID_u8)
  Index/1 -> [ID], Index Vector/1

== system TosAeb/AEB_Objsel/TA_Processor/PreProcessor/ObjectPrecessor/TAObjInfoCalc/For Each Subsystem/Info_Check1 ==
  Obs_Infomation : Inport
  VehSelfIn : Inport  Port=2
  BasicInfo_check : Subsystem  Commented=on
  Basic_Info_check : Subsystem
  Bus Selector : BusSelector  OutputSignals=ObsID_u8,ObsValid_u8,ObsAge_u16,ObsConfidence_u8
  Bus Selector1 : BusSelector  OutputSignals=ObsID_u8,ObsMotionCategory_u8,ObsMotionStatus_u8,ObsClass_u8,ObsVhSubType_u8,ObsPedSubType_u8,ObsPedPos_u8,ObsLength_s,ObsWidth_s,ObsVelXAbs_s,ObsVelYAbs_s
  Bus Selector2 : BusSelector  OutputSignals=ObsID_u8,ObsMotionCategory_u8,ObsPedOrientation_u8,ObsPosX_s,ObsPosY_s,ObsVelY_s,ObsVelX_s,ObsVelXAbs_s,ObsVelYAbs_s,ObsCutInFlg_u8,ObsCutInSpd_s,ObsCutInSpdValid_b,ObsAngle_s,ObsPosType_u8
  Bus Selector3 : BusSelector  OutputSignals=ObsID_u8,ObsMotionStatus_u8,ObsVelXAbs_s,ObsVelYAbs_s
  Bus Selector4 : BusSelector  OutputSignals=ObsID_u8,ObsLaneAssign_u8,ObsPosX_s,ObsPosY_s
  Bus Selector5 : BusSelector  OutputSignals=ObsID_u8,ObsConfidence_u8,ObsPosX_s,ObsPosY_s,ObsVelX_s,ObsVelY_s,ObsAccX_s,ObsPosXSTD_s,ObsPosYSTD_s,ObsVelXSTD_s,ObsVelYSTD_s,ObsAccY_s,ObsVelXAbs_s,ObsVelYAbs_s,ObsAngle_s,ObsAngleSTD_s
  Bus Selector6 : BusSelector  OutputSignals=AEB_Intervention_ESP,ABS_VehicleSpeed,YawRate,AX1,AY1
  Bus Selector7 : BusSelector  OutputSignals=ObsID_u8,ObsLength_s,ObsWidth_s,ObsHeight_s
  Bus Selector8 : BusSelector  OutputSignals=ObsPosXSTD_s,ObsPosYSTD_s,ObsVelXSTD_s,ObsVelYSTD_s,ObsAngleSTD_s,ObsCenterAngle_s,ObsAccX_s,ObsAccY_s
  Dummy_Trans : Subsystem
  FusType : Subsystem
  ID_check : Subsystem
  LaneAssign_check : Subsystem
  Orientation_check : Subsystem
  State_check : Subsystem
  Type_Precheck : Subsystem
  Type_Process : Subsystem
  Fus_ObjID : Outport
  Fus_ObjOrientation : Outport  Port=2
  Fus_ObjState : Outport  Port=3
  Fus_ObjfusType : Outport  Port=4
  Fus_ObjLaneAssign : Outport  Port=5
  Fus_ObjDy : Outport  Port=6
  Fus_ObjDx : Outport  Port=7
  Fus_ObjVx : Outport  Port=8
  Fus_ObjVy : Outport  Port=9
  Fus_ObjQuality : Outport  Port=10
  Fus_ObjAy : Outport  Port=11
  Fus_ObjAx : Outport  Port=12
  Fus_ObjVxAbs : Outport  Port=13
  Fus_ObjVyAbs : Outport  Port=14
  Fus_ObjHeadAngle : Outport  Port=15
  Fus_ObjType : Outport  Port=16
  Fus_ObjLength : Outport  Port=17
  Fus_ObjWidth : Outport  Port=18
  Fus_ObjHight : Outport  Port=19
  Fus_dxSTD : Outport  Port=20
  Fus_dySTD : Outport  Port=21
  Fus_VelxSTD : Outport  Port=22
  Fus_VelySTD : Outport  Port=23
  Fus_HeadAngleSTD : Outport  Port=24
  Fus_CenterAngle : Outport  Port=25
  Fus_AxAbs : Outport  Port=26
  Fus_AyAbs : Outport  Port=27
  wires:
  State_check/1(Fus_ObjState) -> [Fus_ObjState], Fus_ObjState/1
  [Fus_ObsType] -> State_check/2(Fus_ObsType)
  Bus Selector3/3 -> State_check/5(ObsVelXAbs_s)  "<ObsVelXAbs_s>"
  Bus Selector/3 -> ID_check/3(ObsAge_u16)  "<ObsAge_u16>"
  Bus Selector3/1 -> State_check/3(ObsID_u8)  "<ObsID_u8>"
  Bus Selector3/2 -> State_check/4(ObsMeasureStatus_u8)  "<ObsMotionStatus_u8>"
  Bus Selector3/4 -> State_check/6(ObsVelYAbs_s)  "<ObsVelYAbs_s>"
  Bus Selector2/1 -> Orientation_check/4(ObsID_u8)  "<ObsID_u8>"
  [Obs_Infomation] -> Bus Selector3/1
  Bus Selector1/11 -> Type_Precheck/12(ObsVelYAbs_s)  "<ObsVelYAbs_s>"
  ID_check/2(ID_Change) -> [ID_Change]
  Bus Selector2/14 -> Orientation_check/17(ObsPosType_u8)  "<ObsPosType_u8>"
  Bus Selector2/3 -> Orientation_check/6(ObsPedOrientation_u8)  "<ObsPedOrientation_u8>"
  [Obs_Infomation] -> Bus Selector2/1
  Bus Selector2/12 -> Orientation_check/15(ObsCutInSpdValid_b)  "<ObsCutInSpdValid_b>"
  Orientation_check/1(Fus_ObjOrientation) -> Fus_ObjOrientation/1  "Fus_ObjOrientation"
  Bus Selector2/11 -> Orientation_check/14(ObsCutInSpd_s)  "<ObsCutInSpd_s>"
  Bus Selector1/5 -> Type_Precheck/6(ObsVhSubType_u8)  "<ObsVhSubType_u8>"
  Bus Selector1/6 -> Type_Precheck/7(ObsPedSubType_u8)  "<ObsPedSubType_u8>"
  Bus Selector1/4 -> Type_Precheck/5(ObsClass_u8)  "<ObsClass_u8>"
  Bus Selector1/7 -> Type_Precheck/8(ObsPedPos_u8)  "<ObsPedPos_u8>"
  [Obs_Infomation] -> Bus Selector1/1
  Bus Selector2/8 -> Orientation_check/11(ObsVelXAbs_s)  "<ObsVelXAbs_s>"
  Type_Precheck/1(Fus_ObType) -> [Fus_ObsType]  "Fus_ObsType"
  ID_check/1(Fus_ObjID) -> Fus_ObjID/1
  Bus Selector1/3 -> Type_Precheck/4(ObsMotionStatus_u8)  "<ObsMotionStatus_u8>"
  Bus Selector1/9 -> Type_Precheck/10(ObsWidth_s)  "<ObsWidth_s>"
  [ID_Change] -> Type_Precheck/1(ID_Change)
  Bus Selector1/1 -> Type_Precheck/2(ObsID_u8)  "<ObsID_u8>"
  [ID_Change] -> State_check/1(ID_Change)
  Bus Selector2/4 -> Orientation_check/7(ObsPosX_s)  "<ObsPosX_s>"
  Bus Selector1/10 -> Type_Precheck/11(ObsVelXAbs_s)  "<ObsVelXAbs_s>"
  Bus Selector/1 -> ID_check/1(ObsID_u8)  "<ObsID_u8>"
  Bus Selector2/13 -> Orientation_check/16(ObsAngle_s)  "<ObsAngle_s>"
  Bus Selector1/8 -> Type_Precheck/9(ObsLength_s)  "<ObsLength_s>"
  Bus Selector2/5 -> Orientation_check/8(ObsPosY_s)  "<ObsPosY_s>"
  Bus Selector2/10 -> Orientation_check/13(ObsCutInFlg_u8)  "<ObsCutInFlg_u8>"
  Bus Selector/4 -> ID_check/4(ObsConfidence_u8)  "<ObsConfidence_u8>"
  Obs_Infomation/1 -> [Obs_Infomation], Bus Selector/1
  Bus Selector2/9 -> Orientation_check/12(ObsVelYAbs_s)  "<ObsVelYAbs_s>"
  Bus Selector/2 -> ID_check/2(ObsValid_u8)  "<ObsValid_u8>"
  Bus Selector2/2 -> Orientation_check/5(ObsMotionCategory_u8)  "<ObsMotionCategory_u8>"
  Bus Selector1/2 -> Type_Precheck/3(ObsMotionCategory_u8)  "<ObsMotionCategory_u8>"
  FusType/1(Fus_ObjfusType) -> Fus_ObjfusType/1
  [Obs_Infomation] -> Bus Selector4/1
  [ID_Change] -> LaneAssign_check/1(ID_Change)
  Bus Selector4/4 -> LaneAssign_check/5("ObsPosY_s ")  "<ObsPosY_s>"
  Bus Selector4/3 -> LaneAssign_check/4(ObsPosX_s)  "<ObsPosX_s>"
  Bus Selector4/2 -> LaneAssign_check/3(ObsLaneAssign_u8)  "<ObsLaneAssign_u8>"
  Bus Selector4/1 -> LaneAssign_check/2(ObsID_u8)  "<ObsID_u8>"
  LaneAssign_check/1(Fus_ObjLaneAssign) -> Fus_ObjLaneAssign/1
  VehSelfIn/1 -> [VehSelfIn]
  [Obs_Infomation] -> Bus Selector5/1
  [VehSelfIn] -> Bus Selector6/1
  Bus Selector5/7 -> Basic_Info_check/10(ObsAccX_s)  "<ObsAccX_s>"
  Bus Selector5/6 -> Basic_Info_check/9(ObsVelY_s)  "<ObsVelY_s>"
  Bus Selector5/8 -> Basic_Info_check/11(ObsPosXSTD_s)  "<ObsPosXSTD_s>"
  Bus Selector5/5 -> Basic_Info_check/8(ObsVelX_s)  "<ObsVelX_s>"
  Bus Selector5/9 -> Basic_Info_check/12(ObsPosYSTD_s)  "<ObsPosYSTD_s>"
  Bus Selector5/4 -> Basic_Info_check/7(ObsPosY_s)  "<ObsPosY_s>"
  Bus Selector5/10 -> Basic_Info_check/13(ObsVelXSTD_s)  "<ObsVelXSTD_s>"
  Bus Selector5/3 -> Basic_Info_check/6(ObsPosX_s)  "<ObsPosX_s>"
  Bus Selector5/11 -> Basic_Info_check/14(ObsVelYSTD_s)  "<ObsVelYSTD_s>"
  Bus Selector5/12 -> Basic_Info_check/15(ObsAccY_s)  "<ObsAccY_s>"
  Bus Selector5/13 -> Basic_Info_check/16(ObsVelXAbs_s)  "<ObsVelXAbs_s>"
  Bus Selector5/14 -> Basic_Info_check/17(ObsVelYAbs_s)  "<ObsVelYAbs_s>"
  Bus Selector6/3 -> Basic_Info_check/22(Veh_YawRate)  "<YawRate>"
  Bus Selector6/4 -> Basic_Info_check/23(Veh_DrvAcc)  "<AX1>"
  Bus Selector6/2 -> Basic_Info_check/21(Vhe_Spd)  "<ABS_VehicleSpeed>"
  Bus Selector6/5 -> Basic_Info_check/24(Veh_DrvAy)  "<AY1>"
  Bus Selector6/1 -> Basic_Info_check/20(Veh_AEBState)  "<AEB_Intervention_ESP>"
  [ID_Change] -> Basic_Info_check/1(ID_change)
  Bus Selector5/1 -> Basic_Info_check/4(ObsID_u8)  "<ObsID_u8>"
  [Fus_ObjState] -> Basic_Info_check/2(Fus_ObjState)
  Bus Selector5/2 -> Basic_Info_check/5(ObsConfidence_u8)  "<ObsConfidence_u8>"
  [Fus_ObsType] -> Basic_Info_check/3(Fus_Type)
  Bus Selector5/16 -> Basic_Info_check/19(ObsAngleSTD_s)  "<ObsAngleSTD_s>"
  Bus Selector5/15 -> Basic_Info_check/18(ObsAngle_s)  "<ObsAngle_s>"
  Basic_Info_check/1(Fus_ObjDy) -> Fus_ObjDy/1
  Basic_Info_check/2(Fus_ObjDx) -> [Fus_ObjDx], Fus_ObjDx/1
  Basic_Info_check/3(Fus_ObjVx) -> Fus_ObjVx/1
  Basic_Info_check/4(Fus_ObjVy) -> Fus_ObjVy/1
  Basic_Info_check/5(Fus_ObjQuality) -> Fus_ObjQuality/1
  Basic_Info_check/6(Fus_ObjAy) -> Fus_ObjAy/1
  Basic_Info_check/7(Fus_ObjAx) -> Fus_ObjAx/1
  Basic_Info_check/8(Fus_ObjVxAbs) -> [Fus_ObjVxAbs], Fus_ObjVxAbs/1
  Basic_Info_check/9(Fus_ObjVyAbs) -> Fus_ObjVyAbs/1
  Basic_Info_check/10(Fus_ObjHeadAngle) -> Fus_ObjHeadAngle/1
  [Fus_ObsType] -> Type_Process/6(Obj_type)
  [Fus_ObjVxAbs] -> Type_Process/7(abs_Spd)
  [Obs_Infomation] -> Bus Selector7/1
  Bus Selector7/4 -> Type_Process/4(Obj_Height)  "<ObsHeight_s>"
  Bus Selector7/3 -> Type_Process/3(Obj_Width)  "<ObsWidth_s>"
  Bus Selector7/2 -> Type_Process/2(Obj_Length)  "<ObsLength_s>"
  [Fus_ObjDx] -> Type_Process/5(Obj_dx)
  Type_Process/1(Fus_ObjType) -> Fus_ObjType/1
  Type_Process/2(Fus_ObjLength) -> Fus_ObjLength/1
  Type_Process/3(Fus_ObjWidth) -> Fus_ObjWidth/1
  Type_Process/4(Fus_ObjHight) -> Fus_ObjHight/1
  [Obs_Infomation] -> Bus Selector8/1
  Bus Selector8/3 -> Dummy_Trans/3(ObsVelXSTD_s)  "<ObsVelXSTD_s>"
  Bus Selector8/2 -> Dummy_Trans/2(ObsPosYSTD_s)  "<ObsPosYSTD_s>"
  Bus Selector8/4 -> Dummy_Trans/4(ObsVelYSTD_s)  "<ObsVelYSTD_s>"
  Bus Selector8/1 -> Dummy_Trans/1(ObsPosXSTD_s)  "<ObsPosXSTD_s>"
  Bus Selector8/5 -> Dummy_Trans/5(ObsAngleSTD_s)  "<ObsAngleSTD_s>"
  Bus Selector8/6 -> Dummy_Trans/6(ObsCenterAngle_s)  "<ObsCenterAngle_s>"
  Dummy_Trans/2(Fus_dySTD) -> Fus_dySTD/1
  Dummy_Trans/1(Fus_dxSTD) -> Fus_dxSTD/1
  Dummy_Trans/4(Fus_VelySTD) -> Fus_VelySTD/1
  Dummy_Trans/3(Fus_VelxSTD) -> Fus_VelxSTD/1
  Dummy_Trans/5(Fus_HeadAngleSTD) -> Fus_HeadAngleSTD/1
  Dummy_Trans/6(Fus_CenterAngle) -> Fus_CenterAngle/1
  Bus Selector8/7 -> Dummy_Trans/7(ObsAccX_s)  "<ObsAccX_s>"
  Bus Selector8/8 -> Dummy_Trans/8(ObsAccY_s)  "<ObsAccY_s>"
  Dummy_Trans/8(Fus_AyAbs) -> Fus_AyAbs/1
  Dummy_Trans/7(Fus_AxAbs) -> Fus_AxAbs/1
  Bus Selector7/1 -> Type_Process/1(Obj_ID)  "<ObsID_u8>"
  [Fus_ObsType] -> Orientation_check/2(Fus_ObsType)
  [ID_Change] -> Orientation_check/1(ID_Change)
  [Fus_ObjState] -> Orientation_check/3(Obj_Movingstate)
  Bus Selector2/6 -> Orientation_check/9(ObsVelY_s)  "<ObsVelY_s>"
  Bus Selector2/7 -> Orientation_check/10(ObsVelX_s)  "<ObsVelX_s>"

== system TosAeb/AEB_Objsel/TA_Processor/PreProcessor/VehiclePreProcessor/CalculateCurvature/LowPassFilter/LP1_1 ==
  note: y_n-1
  note: y_(n) = w*T*(u_n - y_(n-1)) - y_(n-1)
  U : Inport
      settings: AttributesFormatString=DT=%<OutDataTypeStr>
  TsOverTau : Inport  Port=2
      settings: AttributesFormatString=DT=%<OutDataTypeStr>
  S16_Lag_Filter : Subsystem
  UD_1 : UnitDelay  Description=_3p1p0.CLib.000_109_  SampleTime=-1  HasFrameUpgradeWarning=on
  Y : Outport
      settings: AttributesFormatString=DT=%<OutDataTypeStr>
  wires:
  S16_Lag_Filter/1(filtered_output) -> Y/1, UD_1/1  "Y"
  U/1 -> S16_Lag_Filter/1(unfiltered_input)  "U"
  TsOverTau/1 -> S16_Lag_Filter/3(filter_gain)  "TsOverTau"
  UD_1/1 -> S16_Lag_Filter/2(filtered_value_n_1)  "LastY"
